simulink model "large_model"  (Simulink 8.0)
settings: Solver=ode45  StartTime=0.0  StopTime=10.0  RelTol=1e-3
(part 2 of 21)

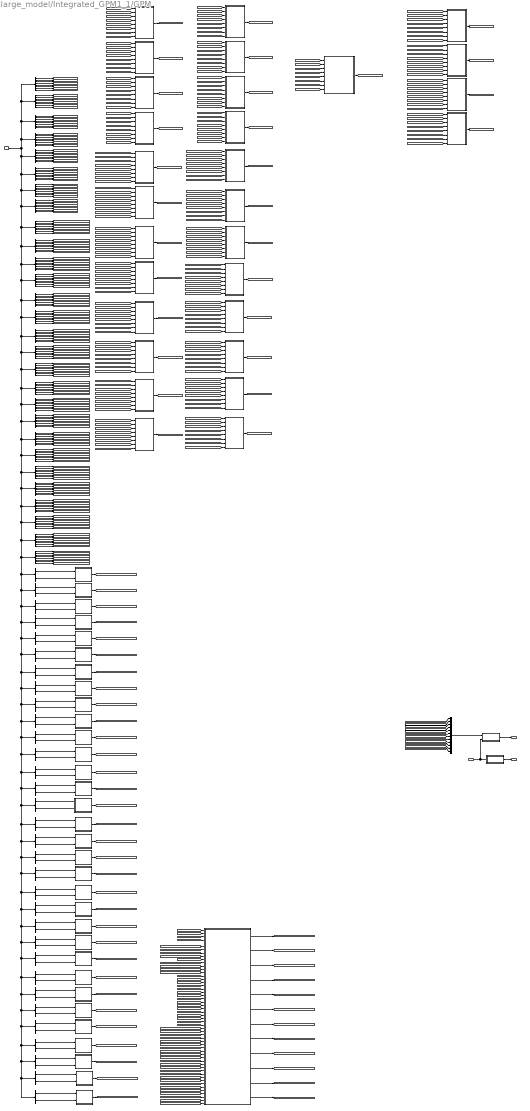
== system large_model/Integrated_GPM1_1/GPM ==
  note: Este componente foi colocado devido a uma limitação do EFTG. Na versão 1.2.1,
      | não é permitido fazer uma conexão direta entre a entrada e a saída.
  From_ES : Inport
  PWR_In : Inport  Port=2
  CAB1_FCC1 : Subsystem
  CAB1_GPM2_fcc_ram_mon_AIL_LIB_dat : BusSelector  OutputSignals=switch1A.cab1_gpm2_chB.CAB1_GPM2_fcc_ram_mon_AIL_LIB_dat,switch1B.cab1_gpm2_chB.CAB1_GPM2_fcc_ram_mon_AIL_LIB_dat
  CAB1_GPM2_fcc_ram_mon_AIL_LOB_dat : BusSelector  OutputSignals=switch1A.cab1_gpm2_chB.CAB1_GPM2_fcc_ram_mon_AIL_LOB_dat,switch1B.cab1_gpm2_chB.CAB1_GPM2_fcc_ram_mon_AIL_LOB_dat
  CAB1_GPM2_fcc_ram_mon_AIL_RIB_dat : BusSelector  OutputSignals=switch1A.cab1_gpm2_chB.CAB1_GPM2_fcc_ram_mon_AIL_RIB_dat,switch1B.cab1_gpm2_chB.CAB1_GPM2_fcc_ram_mon_AIL_RIB_dat
  CAB1_GPM2_fcc_ram_mon_AIL_ROB_dat : BusSelector  OutputSignals=switch1A.cab1_gpm2_chB.CAB1_GPM2_fcc_ram_mon_AIL_ROB_dat,switch1B.cab1_gpm2_chB.CAB1_GPM2_fcc_ram_mon_AIL_ROB_dat
  CAB1_GPM2_fcc_ram_mon_ELEV_LIB_dat : BusSelector  OutputSignals=switch1A.cab1_gpm2_chB.CAB1_GPM2_fcc_ram_mon_ELEV_LIB_dat,switch1B.cab1_gpm2_chB.CAB1_GPM2_fcc_ram_mon_ELEV_LIB_dat
  CAB1_GPM2_fcc_ram_mon_ELEV_LOB_dat : BusSelector  OutputSignals=switch1A.cab1_gpm2_chB.CAB1_GPM2_fcc_ram_mon_ELEV_LOB_dat,switch1B.cab1_gpm2_chB.CAB1_GPM2_fcc_ram_mon_ELEV_LOB_dat
  CAB1_GPM2_fcc_ram_mon_ELEV_RIB_dat : BusSelector  OutputSignals=switch1A.cab1_gpm2_chB.CAB1_GPM2_fcc_ram_mon_ELEV_RIB_dat,switch1B.cab1_gpm2_chB.CAB1_GPM2_fcc_ram_mon_ELEV_RIB_dat
  CAB1_GPM2_fcc_ram_mon_ELEV_ROB_dat : BusSelector  OutputSignals=switch1A.cab1_gpm2_chB.CAB1_GPM2_fcc_ram_mon_ELEV_ROB_dat,switch1B.cab1_gpm2_chB.CAB1_GPM2_fcc_ram_mon_ELEV_ROB_dat
  CAB1_GPM2_fcc_ram_mon_rudder_LWR_dat : BusSelector  OutputSignals=switch1A.cab1_gpm2_chB.CAB1_GPM2_fcc_ram_mon_rudder_LWR_dat,switch1B.cab1_gpm2_chB.CAB1_GPM2_fcc_ram_mon_rudder_LWR_dat
  CAB1_GPM2_fcc_ram_mon_rudder_UPR_dat : BusSelector  OutputSignals=switch1A.cab1_gpm2_chB.CAB1_GPM2_fcc_ram_mon_rudder_UPR_dat,switch1B.cab1_gpm2_chB.CAB1_GPM2_fcc_ram_mon_rudder_UPR_dat
  CAB1_GPM2_pitch_roll_cmd : BusSelector  OutputSignals=switch1A.cab1_gpm2_chB.CAB1_GPM2_pitch_roll_cmd,switch1B.cab1_gpm2_chB.CAB1_GPM2_pitch_roll_cmd
  CAB1_GPM2_yaw_cmd : BusSelector  OutputSignals=switch1A.cab1_gpm2_chB.CAB1_GPM2_yaw_cmd,switch1B.cab1_gpm2_chB.CAB1_GPM2_yaw_cmd
  GPM Hardware : Subsystem
  Outputs : BusCreator  Inputs=12
  PWR : Subsystem
  SCP_CAB1_GPM2_fcc_ram_mon_AIL_LIB : Subsystem
  SCP_CAB1_GPM2_fcc_ram_mon_AIL_LOB : Subsystem
  SCP_CAB1_GPM2_fcc_ram_mon_AIL_RIB : Subsystem
  SCP_CAB1_GPM2_fcc_ram_mon_AIL_ROB : Subsystem
  SCP_CAB1_GPM2_fcc_ram_mon_ELEV_LIB : Subsystem
  SCP_CAB1_GPM2_fcc_ram_mon_ELEV_LOB : Subsystem
  SCP_CAB1_GPM2_fcc_ram_mon_ELEV_RIB : Subsystem
  SCP_CAB1_GPM2_fcc_ram_mon_ELEV_ROB : Subsystem
  SCP_CAB1_GPM2_fcc_ram_mon_rudder_LWR : Subsystem
  SCP_CAB1_GPM2_fcc_ram_mon_rudder_UPR : Subsystem
  SCP_CAB1_GPM2_pitch_roll : Subsystem
  SCP_CAB1_GPM2_yaw : Subsystem
  SCP_ail_REU_LIB_LVDT : Subsystem
  SCP_ail_REU_LIB_mon : Subsystem
  SCP_ail_REU_LOB_LVDT : Subsystem
  SCP_ail_REU_LOB_mon : Subsystem
  SCP_ail_REU_RIB_LVDT : Subsystem
  SCP_ail_REU_RIB_mon : Subsystem
  SCP_ail_REU_ROB_LVDT : Subsystem
  SCP_ail_REU_ROB_mon : Subsystem
  SCP_elev_REU_LIB_LVDT : Subsystem
  SCP_elev_REU_LIB_mon_dat : Subsystem
  SCP_elev_REU_LOB_LVDT : Subsystem
  SCP_elev_REU_LOB_mon : Subsystem
  SCP_elev_REU_RIB_LVDT : Subsystem
  SCP_elev_REU_RIB_mon : Subsystem
  SCP_elev_REU_ROB_LVDT : Subsystem
  SCP_elev_REU_ROB_mon : Subsystem
  SCP_rud_REU1_LVDT : Subsystem
  SCP_rud_REU1_MON : Subsystem
  SCP_rud_REU2_LVDT : Subsystem
  SCP_rud_REU2_MON : Subsystem
  Self_checking_pair_ADS1A : Subsystem
  Self_checking_pair_ADS1B : Subsystem
  Self_checking_pair_ADS2A : Subsystem
  Self_checking_pair_ADS2B : Subsystem
  Self_checking_pair_ADS3A : Subsystem
  Self_checking_pair_ADS3B : Subsystem
  Self_checking_pair_ADS4A : Subsystem
  Self_checking_pair_ADS4B : Subsystem
  Self_checking_pair_PedalYaw1 : Subsystem
  Self_checking_pair_PedalYaw2 : Subsystem
  Self_checking_pair_PedalYaw3 : Subsystem
  Self_checking_pair_PedalYaw4 : Subsystem
  Self_checking_pair_SSC1_pitch1 : Subsystem
  Self_checking_pair_SSC1_pitch2 : Subsystem
  Self_checking_pair_SSC1_pitch3 : Subsystem
  Self_checking_pair_SSC1_pitch4 : Subsystem
  Self_checking_pair_SSC1_roll1 : Subsystem
  Self_checking_pair_SSC1_roll2 : Subsystem
  Self_checking_pair_SSC1_roll3 : Subsystem
  Self_checking_pair_SSC1_roll4 : Subsystem
  Self_checking_pair_SSC2_pitch1 : Subsystem
  Self_checking_pair_SSC2_pitch2 : Subsystem
  Self_checking_pair_SSC2_pitch3 : Subsystem
  Self_checking_pair_SSC2_pitch4 : Subsystem
  Self_checking_pair_SSC2_roll1 : Subsystem
  Self_checking_pair_SSC2_roll2 : Subsystem
  Self_checking_pair_SSC2_roll3 : Subsystem
  Self_checking_pair_SSC2_roll4 : Subsystem
  Voter_ADS : Subsystem
  ads_1A : BusSelector  Description=|  OutputSignals=switch1A.cab2_gpm_IO_chB.ads_1A,switch1A.cab1_gpm_IO_chB.ads_1A,switch1A.switch2A_to_switch.cab3_gpm_IO_chA.ads_1A,switch1A.switch2A_to_switch.cab4_gpm_IO_chA.ads_1A,switch1B.cab2_gpm_IO_chB.ads_1A,switch1B.cab1_gpm_IO_chB.ads_1A,switch1B.switch2B_to_switch.cab4_gpm_IO_chB.ads_1A,switch1B.switch2B_to_switch.cab3_gpm_IO_chB.ads_1A
      | When connected to a line which contains bus signals, this bus selector will select as its outputs all the signals which contain this bus selector name.
      | For example, if you name this bus selector as "sidestick_1_pitch_1", all signals containing this string will be selected as its outputs.
  ads_1B : BusSelector  Description=|  OutputSignals=switch1A.cab2_gpm_IO_chB.ads_1B,switch1A.cab1_gpm_IO_chB.ads_1B,switch1A.switch2A_to_switch.cab3_gpm_IO_chA.ads_1B,switch1A.switch2A_to_switch.cab4_gpm_IO_chA.ads_1B,switch1B.cab2_gpm_IO_chB.ads_1B,switch1B.cab1_gpm_IO_chB.ads_1B,switch1B.switch2B_to_switch.cab4_gpm_IO_chB.ads_1B,switch1B.switch2B_to_switch.cab3_gpm_IO_chB.ads_1B
      | When connected to a line which contains bus signals, this bus selector will select as its outputs all the signals which contain this bus selector name.
      | For example, if you name this bus selector as "sidestick_1_pitch_1", all signals containing this string will be selected as its outputs.
  ads_2A : BusSelector  Description=|  OutputSignals=switch1A.cab2_gpm_IO_chB.ads_2A,switch1A.cab1_gpm_IO_chB.ads_2A,switch1A.switch2A_to_switch.cab3_gpm_IO_chA.ads_2A,switch1A.switch2A_to_switch.cab4_gpm_IO_chA.ads_2A,switch1B.cab2_gpm_IO_chB.ads_2A,switch1B.cab1_gpm_IO_chB.ads_2A,switch1B.switch2B_to_switch.cab4_gpm_IO_chB.ads_2A,switch1B.switch2B_to_switch.cab3_gpm_IO_chB.ads_2A
      | When connected to a line which contains bus signals, this bus selector will select as its outputs all the signals which contain this bus selector name.
      | For example, if you name this bus selector as "sidestick_1_pitch_1", all signals containing this string will be selected as its outputs.
  ads_2B : BusSelector  Description=|  OutputSignals=switch1A.cab2_gpm_IO_chB.ads_2B,switch1A.cab1_gpm_IO_chB.ads_2B,switch1A.switch2A_to_switch.cab3_gpm_IO_chA.ads_2B,switch1A.switch2A_to_switch.cab4_gpm_IO_chA.ads_2B,switch1B.cab2_gpm_IO_chB.ads_2B,switch1B.cab1_gpm_IO_chB.ads_2B,switch1B.switch2B_to_switch.cab4_gpm_IO_chB.ads_2B,switch1B.switch2B_to_switch.cab3_gpm_IO_chB.ads_2B
      | When connected to a line which contains bus signals, this bus selector will select as its outputs all the signals which contain this bus selector name.
      | For example, if you name this bus selector as "sidestick_1_pitch_1", all signals containing this string will be selected as its outputs.
  ads_3A : BusSelector  Description=|  OutputSignals=switch1A.cab2_gpm_IO_chB.ads_3A,switch1A.cab1_gpm_IO_chB.ads_3A,switch1A.switch2A_to_switch.cab3_gpm_IO_chA.ads_3A,switch1A.switch2A_to_switch.cab4_gpm_IO_chA.ads_3A,switch1B.cab2_gpm_IO_chB.ads_3A,switch1B.cab1_gpm_IO_chB.ads_3A,switch1B.switch2B_to_switch.cab4_gpm_IO_chB.ads_3A,switch1B.switch2B_to_switch.cab3_gpm_IO_chB.ads_3A
      | When connected to a line which contains bus signals, this bus selector will select as its outputs all the signals which contain this bus selector name.
      | For example, if you name this bus selector as "sidestick_1_pitch_1", all signals containing this string will be selected as its outputs.
  ads_3B : BusSelector  Description=|  OutputSignals=switch1A.cab2_gpm_IO_chB.ads_3B,switch1A.cab1_gpm_IO_chB.ads_3B,switch1A.switch2A_to_switch.cab3_gpm_IO_chA.ads_3B,switch1A.switch2A_to_switch.cab4_gpm_IO_chA.ads_3B,switch1B.cab2_gpm_IO_chB.ads_3B,switch1B.cab1_gpm_IO_chB.ads_3B,switch1B.switch2B_to_switch.cab4_gpm_IO_chB.ads_3B,switch1B.switch2B_to_switch.cab3_gpm_IO_chB.ads_3B
      | When connected to a line which contains bus signals, this bus selector will select as its outputs all the signals which contain this bus selector name.
      | For example, if you name this bus selector as "sidestick_1_pitch_1", all signals containing this string will be selected as its outputs.
  ads_4A : BusSelector  Description=|  OutputSignals=switch1A.cab2_gpm_IO_chB.ads_4A,switch1A.cab1_gpm_IO_chB.ads_4A,switch1A.switch2A_to_switch.cab3_gpm_IO_chA.ads_4A,switch1A.switch2A_to_switch.cab4_gpm_IO_chA.ads_4A,switch1B.cab2_gpm_IO_chB.ads_4A,switch1B.cab1_gpm_IO_chB.ads_4A,switch1B.switch2B_to_switch.cab4_gpm_IO_chB.ads_4A,switch1B.switch2B_to_switch.cab3_gpm_IO_chB.ads_4A
      | When connected to a line which contains bus signals, this bus selector will select as its outputs all the signals which contain this bus selector name.
      | For example, if you name this bus selector as "sidestick_1_pitch_1", all signals containing this string will be selected as its outputs.
  ads_4B : BusSelector  Description=|  OutputSignals=switch1A.cab2_gpm_IO_chB.ads_4B,switch1A.cab1_gpm_IO_chB.ads_4B,switch1A.switch2A_to_switch.cab3_gpm_IO_chA.ads_4B,switch1A.switch2A_to_switch.cab4_gpm_IO_chA.ads_4B,switch1B.cab2_gpm_IO_chB.ads_4B,switch1B.cab1_gpm_IO_chB.ads_4B,switch1B.switch2B_to_switch.cab4_gpm_IO_chB.ads_4B,switch1B.switch2B_to_switch.cab3_gpm_IO_chB.ads_4B
      | When connected to a line which contains bus signals, this bus selector will select as its outputs all the signals which contain this bus selector name.
      | For example, if you name this bus selector as "sidestick_1_pitch_1", all signals containing this string will be selected as its outputs.
  ail_REU_LIB_LVDT_dat : BusSelector  OutputSignals=switch1A.switch2A_to_switch.REU_to_Switch2A.ail_REU_LIB_LVDT_dat,switch1B.switch2B_to_switch.REU_to_Switch2B.ail_REU_LIB_LVDT_dat
  ail_REU_LIB_mon_dat : BusSelector  OutputSignals=switch1A.switch2A_to_switch.REU_to_Switch2A.ail_REU_LIB_mon_dat,switch1B.switch2B_to_switch.REU_to_Switch2B.ail_REU_LIB_mon_dat
  ail_REU_LOB_LVDT_dat : BusSelector  OutputSignals=switch1A.REU_to_Switch1A.ail_REU_LOB_LVDT_dat,switch1B.REU_to_Switch1B.ail_REU_LOB_LVDT_dat
  ail_REU_LOB_mon_dat : BusSelector  OutputSignals=switch1A.REU_to_Switch1A.ail_REU_LOB_mon_dat,switch1B.REU_to_Switch1B.ail_REU_LOB_mon_dat
  ail_REU_RIB_LVDT_dat : BusSelector  OutputSignals=switch1A.switch2A_to_switch.REU_to_Switch2A.ail_REU_RIB_LVDT_dat,switch1B.switch2B_to_switch.REU_to_Switch2B.ail_REU_RIB_LVDT_dat
  ail_REU_RIB_mon_dat : BusSelector  OutputSignals=switch1A.switch2A_to_switch.REU_to_Switch2A.ail_REU_RIB_mon_dat,switch1B.switch2B_to_switch.REU_to_Switch2B.ail_REU_RIB_mon_dat
  ail_REU_ROB_LVDT_dat : BusSelector  OutputSignals=switch1A.REU_to_Switch1A.ail_REU_ROB_LVDT_dat,switch1B.REU_to_Switch1B.ail_REU_ROB_LVDT_dat
  ail_REU_ROB_mon_dat : BusSelector  OutputSignals=switch1A.REU_to_Switch1A.ail_REU_ROB_mon_dat,switch1B.REU_to_Switch1B.ail_REU_ROB_mon_dat
  elev_REU_LIB_LVDT_dat : BusSelector  OutputSignals=switch1A.switch2A_to_switch.REU_to_Switch2A.elev_REU_LIB_LVDT_dat,switch1B.switch2B_to_switch.REU_to_Switch2B.elev_REU_LIB_LVDT_dat
  elev_REU_LIB_mon_dat : BusSelector  OutputSignals=switch1A.switch2A_to_switch.REU_to_Switch2A.elev_REU_LIB_mon_dat,switch1B.switch2B_to_switch.REU_to_Switch2B.elev_REU_LIB_mon_dat
  elev_REU_LOB_LVDT_dat : BusSelector  OutputSignals=switch1A.REU_to_Switch1A.elev_REU_LOB_LVDT_dat,switch1B.REU_to_Switch1B.elev_REU_LOB_LVDT_dat
  elev_REU_LOB_mon_dat : BusSelector  OutputSignals=switch1A.REU_to_Switch1A.elev_REU_LOB_mon_dat,switch1B.REU_to_Switch1B.elev_REU_LOB_mon_dat
  elev_REU_RIB_LVDT_dat : BusSelector  OutputSignals=switch1A.switch2A_to_switch.REU_to_Switch2A.elev_REU_RIB_LVDT_dat,switch1B.switch2B_to_switch.REU_to_Switch2B.elev_REU_RIB_LVDT_dat
  elev_REU_RIB_mon_dat : BusSelector  OutputSignals=switch1A.switch2A_to_switch.REU_to_Switch2A.elev_REU_RIB_mon_dat,switch1B.switch2B_to_switch.REU_to_Switch2B.elev_REU_RIB_mon_dat
  elev_REU_ROB_LVDT_dat : BusSelector  OutputSignals=switch1A.REU_to_Switch1A.elev_REU_ROB_LVDT_dat,switch1B.REU_to_Switch1B.elev_REU_ROB_LVDT_dat
  elev_REU_ROB_mon_dat : BusSelector  OutputSignals=switch1A.REU_to_Switch1A.elev_REU_ROB_mon_dat,switch1B.REU_to_Switch1B.elev_REU_ROB_mon_dat
  pedal_yaw_1 : BusSelector  Description=|  OutputSignals=switch1A.cab2_gpm_IO_chB.rdc_4002_1.pedal_yaw_1,switch1A.cab1_gpm_IO_chB.rdc_4002_1.pedal_yaw_1,switch1A.switch2A_to_switch.cab3_gpm_IO_chA.rdc_4002_1.pedal_yaw_1,switch1A.switch2A_to_switch.cab4_gpm_IO_chA.rdc_4002_1.pedal_yaw_1,switch1B.cab2_gpm_IO_chB.rdc_4002_1.pedal_yaw_1,switch1B.cab1_gpm_IO_chB.rdc_4002_1.pedal_yaw_1,switch1B.switch2B_to_switch.cab4_gpm_IO_chB.rdc_4002_1.pedal_yaw_1,switch1B.switch2B_to_switch.cab3_gpm_IO_chB.rdc_4002_1.pedal_yaw_1
      | When connected to a line which contains bus signals, this bus selector will select as its outputs all the signals which contain this bus selector name.
      | For example, if you name this bus selector as "sidestick_1_pitch_1", all signals containing this string will be selected as its outputs.
  pedal_yaw_2 : BusSelector  Description=|  OutputSignals=switch1A.cab2_gpm_IO_chB.rdc_4002_2.pedal_yaw_2,switch1A.cab1_gpm_IO_chB.rdc_4002_2.pedal_yaw_2,switch1A.switch2A_to_switch.cab3_gpm_IO_chA.rdc_4002_2.pedal_yaw_2,switch1A.switch2A_to_switch.cab4_gpm_IO_chA.rdc_4002_2.pedal_yaw_2,switch1B.cab2_gpm_IO_chB.rdc_4002_2.pedal_yaw_2,switch1B.cab1_gpm_IO_chB.rdc_4002_2.pedal_yaw_2,switch1B.switch2B_to_switch.cab4_gpm_IO_chB.rdc_4002_2.pedal_yaw_2,switch1B.switch2B_to_switch.cab3_gpm_IO_chB.rdc_4002_2.pedal_yaw_2
      | When connected to a line which contains bus signals, this bus selector will select as its outputs all the signals which contain this bus selector name.
      | For example, if you name this bus selector as "sidestick_1_pitch_1", all signals containing this string will be selected as its outputs.
  pedal_yaw_3 : BusSelector  Description=|  OutputSignals=switch1A.cab2_gpm_IO_chB.rdc_4002_3.pedal_yaw_3,switch1A.cab1_gpm_IO_chB.rdc_4002_3.pedal_yaw_3,switch1A.switch2A_to_switch.cab3_gpm_IO_chA.rdc_4002_3.pedal_yaw_3,switch1A.switch2A_to_switch.cab4_gpm_IO_chA.rdc_4002_3.pedal_yaw_3,switch1B.cab2_gpm_IO_chB.rdc_4002_3.pedal_yaw_3,switch1B.cab1_gpm_IO_chB.rdc_4002_3.pedal_yaw_3,switch1B.switch2B_to_switch.cab4_gpm_IO_chB.rdc_4002_3.pedal_yaw_3,switch1B.switch2B_to_switch.cab3_gpm_IO_chB.rdc_4002_3.pedal_yaw_3
      | When connected to a line which contains bus signals, this bus selector will select as its outputs all the signals which contain this bus selector name.
      | For example, if you name this bus selector as "sidestick_1_pitch_1", all signals containing this string will be selected as its outputs.
  pedal_yaw_4 : BusSelector  Description=|  OutputSignals=switch1A.cab2_gpm_IO_chB.rdc_4002_4.pedal_yaw_4,switch1A.cab1_gpm_IO_chB.rdc_4002_4.pedal_yaw_4,switch1A.switch2A_to_switch.cab3_gpm_IO_chA.rdc_4002_4.pedal_yaw_4,switch1A.switch2A_to_switch.cab4_gpm_IO_chA.rdc_4002_4.pedal_yaw_4,switch1B.cab2_gpm_IO_chB.rdc_4002_4.pedal_yaw_4,switch1B.cab1_gpm_IO_chB.rdc_4002_4.pedal_yaw_4,switch1B.switch2B_to_switch.cab4_gpm_IO_chB.rdc_4002_4.pedal_yaw_4,switch1B.switch2B_to_switch.cab3_gpm_IO_chB.rdc_4002_4.pedal_yaw_4
      | When connected to a line which contains bus signals, this bus selector will select as its outputs all the signals which contain this bus selector name.
      | For example, if you name this bus selector as "sidestick_1_pitch_1", all signals containing this string will be selected as its outputs.
  rud_REU1_LVDT_dat : BusSelector  OutputSignals=switch1A.switch2A_to_switch.REU_to_Switch2A.rud_REU1_LVDT_dat,switch1B.switch2B_to_switch.REU_to_Switch2B.rud_REU1_LVDT_dat
  rud_REU1_MON_dat : BusSelector  OutputSignals=switch1A.switch2A_to_switch.REU_to_Switch2A.rud_REU1_MON_dat,switch1B.switch2B_to_switch.REU_to_Switch2B.rud_REU1_MON_dat
  rud_REU2_LVDT_dat : BusSelector  OutputSignals=switch1A.REU_to_Switch1A.rud_REU2_LVDT_dat,switch1B.REU_to_Switch1B.rud_REU2_LVDT_dat
  rud_REU2_MON_dat : BusSelector  OutputSignals=switch1A.REU_to_Switch1A.rud_REU2_MON_dat,switch1B.REU_to_Switch1B.rud_REU2_MON_dat
  sidestick1_pitch_1 : BusSelector  OutputSignals=switch1A.cab2_gpm_IO_chB.rdc_4002_1.sidesticks.sidestick1_pitch_1,switch1A.cab1_gpm_IO_chB.rdc_4002_1.sidesticks.sidestick1_pitch_1,switch1A.switch2A_to_switch.cab3_gpm_IO_chA.rdc_4002_1.sidesticks.sidestick1_pitch_1,switch1A.switch2A_to_switch.cab4_gpm_IO_chA.rdc_4002_1.sidesticks.sidestick1_pitch_1,switch1B.cab2_gpm_IO_chB.rdc_4002_1.sidesticks.sidestick1_pitch_1,switch1B.cab1_gpm_IO_chB.rdc_4002_1.sidesticks.sidestick1_pitch_1,switch1B.switch2B_to_switch.cab4_gpm_IO_chB.rdc_4002_1.sidesticks.sidestick1_pitch_1,switch1B.switch2B_to_switch.cab3_gpm_IO_chB.rdc_4002_1.sidesticks.sidestick1_pitch_1
  sidestick1_pitch_2 : BusSelector  OutputSignals=switch1A.cab2_gpm_IO_chB.rdc_4002_2.sidesticks.sidestick1_pitch_2,switch1A.cab1_gpm_IO_chB.rdc_4002_2.sidesticks.sidestick1_pitch_2,switch1A.switch2A_to_switch.cab3_gpm_IO_chA.rdc_4002_2.sidesticks.sidestick1_pitch_2,switch1A.switch2A_to_switch.cab4_gpm_IO_chA.rdc_4002_2.sidesticks.sidestick1_pitch_2,switch1B.cab2_gpm_IO_chB.rdc_4002_2.sidesticks.sidestick1_pitch_2,switch1B.cab1_gpm_IO_chB.rdc_4002_2.sidesticks.sidestick1_pitch_2,switch1B.switch2B_to_switch.cab4_gpm_IO_chB.rdc_4002_2.sidesticks.sidestick1_pitch_2,switch1B.switch2B_to_switch.cab3_gpm_IO_chB.rdc_4002_2.sidesticks.sidestick1_pitch_2
  sidestick1_pitch_3 : BusSelector  OutputSignals=switch1A.cab2_gpm_IO_chB.rdc_4002_3.sidesticks.sidestick1_pitch_3,switch1A.cab1_gpm_IO_chB.rdc_4002_3.sidesticks.sidestick1_pitch_3,switch1A.switch2A_to_switch.cab3_gpm_IO_chA.rdc_4002_3.sidesticks.sidestick1_pitch_3,switch1A.switch2A_to_switch.cab4_gpm_IO_chA.rdc_4002_3.sidesticks.sidestick1_pitch_3,switch1B.cab2_gpm_IO_chB.rdc_4002_3.sidesticks.sidestick1_pitch_3,switch1B.cab1_gpm_IO_chB.rdc_4002_3.sidesticks.sidestick1_pitch_3,switch1B.switch2B_to_switch.cab4_gpm_IO_chB.rdc_4002_3.sidesticks.sidestick1_pitch_3,switch1B.switch2B_to_switch.cab3_gpm_IO_chB.rdc_4002_3.sidesticks.sidestick1_pitch_3
  sidestick1_pitch_4 : BusSelector  OutputSignals=switch1A.cab2_gpm_IO_chB.rdc_4002_4.sidesticks.sidestick1_pitch_4,switch1A.cab1_gpm_IO_chB.rdc_4002_4.sidesticks.sidestick1_pitch_4,switch1A.switch2A_to_switch.cab3_gpm_IO_chA.rdc_4002_4.sidesticks.sidestick1_pitch_4,switch1A.switch2A_to_switch.cab4_gpm_IO_chA.rdc_4002_4.sidesticks.sidestick1_pitch_4,switch1B.cab2_gpm_IO_chB.rdc_4002_4.sidesticks.sidestick1_pitch_4,switch1B.cab1_gpm_IO_chB.rdc_4002_4.sidesticks.sidestick1_pitch_4,switch1B.switch2B_to_switch.cab4_gpm_IO_chB.rdc_4002_4.sidesticks.sidestick1_pitch_4,switch1B.switch2B_to_switch.cab3_gpm_IO_chB.rdc_4002_4.sidesticks.sidestick1_pitch_4
  sidestick1_roll_1 : BusSelector  OutputSignals=switch1A.cab2_gpm_IO_chB.rdc_4002_1.sidesticks.sidestick1_roll_1,switch1A.cab1_gpm_IO_chB.rdc_4002_1.sidesticks.sidestick1_roll_1,switch1A.switch2A_to_switch.cab3_gpm_IO_chA.rdc_4002_1.sidesticks.sidestick1_roll_1,switch1A.switch2A_to_switch.cab4_gpm_IO_chA.rdc_4002_1.sidesticks.sidestick1_roll_1,switch1B.cab2_gpm_IO_chB.rdc_4002_1.sidesticks.sidestick1_roll_1,switch1B.cab1_gpm_IO_chB.rdc_4002_1.sidesticks.sidestick1_roll_1,switch1B.switch2B_to_switch.cab4_gpm_IO_chB.rdc_4002_1.sidesticks.sidestick1_roll_1,switch1B.switch2B_to_switch.cab3_gpm_IO_chB.rdc_4002_1.sidesticks.sidestick1_roll_1
  sidestick1_roll_2 : BusSelector  OutputSignals=switch1A.cab2_gpm_IO_chB.rdc_4002_2.sidesticks.sidestick1_roll_2,switch1A.cab1_gpm_IO_chB.rdc_4002_2.sidesticks.sidestick1_roll_2,switch1A.switch2A_to_switch.cab3_gpm_IO_chA.rdc_4002_2.sidesticks.sidestick1_roll_2,switch1A.switch2A_to_switch.cab4_gpm_IO_chA.rdc_4002_2.sidesticks.sidestick1_roll_2,switch1B.cab2_gpm_IO_chB.rdc_4002_2.sidesticks.sidestick1_roll_2,switch1B.cab1_gpm_IO_chB.rdc_4002_2.sidesticks.sidestick1_roll_2,switch1B.switch2B_to_switch.cab4_gpm_IO_chB.rdc_4002_2.sidesticks.sidestick1_roll_2,switch1B.switch2B_to_switch.cab3_gpm_IO_chB.rdc_4002_2.sidesticks.sidestick1_roll_2
  sidestick1_roll_3 : BusSelector  OutputSignals=switch1A.cab2_gpm_IO_chB.rdc_4002_3.sidesticks.sidestick1_roll_3,switch1A.cab1_gpm_IO_chB.rdc_4002_3.sidesticks.sidestick1_roll_3,switch1A.switch2A_to_switch.cab3_gpm_IO_chA.rdc_4002_3.sidesticks.sidestick1_roll_3,switch1A.switch2A_to_switch.cab4_gpm_IO_chA.rdc_4002_3.sidesticks.sidestick1_roll_3,switch1B.cab2_gpm_IO_chB.rdc_4002_3.sidesticks.sidestick1_roll_3,switch1B.cab1_gpm_IO_chB.rdc_4002_3.sidesticks.sidestick1_roll_3,switch1B.switch2B_to_switch.cab4_gpm_IO_chB.rdc_4002_3.sidesticks.sidestick1_roll_3,switch1B.switch2B_to_switch.cab3_gpm_IO_chB.rdc_4002_3.sidesticks.sidestick1_roll_3
  sidestick1_roll_4 : BusSelector  OutputSignals=switch1A.cab2_gpm_IO_chB.rdc_4002_4.sidesticks.sidestick1_roll_4,switch1A.cab1_gpm_IO_chB.rdc_4002_4.sidesticks.sidestick1_roll_4,switch1A.switch2A_to_switch.cab3_gpm_IO_chA.rdc_4002_4.sidesticks.sidestick1_roll_4,switch1A.switch2A_to_switch.cab4_gpm_IO_chA.rdc_4002_4.sidesticks.sidestick1_roll_4,switch1B.cab2_gpm_IO_chB.rdc_4002_4.sidesticks.sidestick1_roll_4,switch1B.cab1_gpm_IO_chB.rdc_4002_4.sidesticks.sidestick1_roll_4,switch1B.switch2B_to_switch.cab4_gpm_IO_chB.rdc_4002_4.sidesticks.sidestick1_roll_4,switch1B.switch2B_to_switch.cab3_gpm_IO_chB.rdc_4002_4.sidesticks.sidestick1_roll_4
  sidestick2_pitch_1 : BusSelector  OutputSignals=switch1A.cab2_gpm_IO_chB.rdc_4002_1.sidesticks.sidestick2_pitch_1,switch1A.cab1_gpm_IO_chB.rdc_4002_1.sidesticks.sidestick2_pitch_1,switch1A.switch2A_to_switch.cab3_gpm_IO_chA.rdc_4002_1.sidesticks.sidestick2_pitch_1,switch1A.switch2A_to_switch.cab4_gpm_IO_chA.rdc_4002_1.sidesticks.sidestick2_pitch_1,switch1B.cab2_gpm_IO_chB.rdc_4002_1.sidesticks.sidestick2_pitch_1,switch1B.cab1_gpm_IO_chB.rdc_4002_1.sidesticks.sidestick2_pitch_1,switch1B.switch2B_to_switch.cab4_gpm_IO_chB.rdc_4002_1.sidesticks.sidestick2_pitch_1,switch1B.switch2B_to_switch.cab3_gpm_IO_chB.rdc_4002_1.sidesticks.sidestick2_pitch_1
  sidestick2_pitch_2 : BusSelector  OutputSignals=switch1A.cab2_gpm_IO_chB.rdc_4002_2.sidesticks.sidestick2_pitch_2,switch1A.cab1_gpm_IO_chB.rdc_4002_2.sidesticks.sidestick2_pitch_2,switch1A.switch2A_to_switch.cab3_gpm_IO_chA.rdc_4002_2.sidesticks.sidestick2_pitch_2,switch1A.switch2A_to_switch.cab4_gpm_IO_chA.rdc_4002_2.sidesticks.sidestick2_pitch_2,switch1B.cab2_gpm_IO_chB.rdc_4002_2.sidesticks.sidestick2_pitch_2,switch1B.cab1_gpm_IO_chB.rdc_4002_2.sidesticks.sidestick2_pitch_2,switch1B.switch2B_to_switch.cab4_gpm_IO_chB.rdc_4002_2.sidesticks.sidestick2_pitch_2,switch1B.switch2B_to_switch.cab3_gpm_IO_chB.rdc_4002_2.sidesticks.sidestick2_pitch_2
  sidestick2_pitch_3 : BusSelector  OutputSignals=switch1A.cab2_gpm_IO_chB.rdc_4002_3.sidesticks.sidestick2_pitch_3,switch1A.cab1_gpm_IO_chB.rdc_4002_3.sidesticks.sidestick2_pitch_3,switch1A.switch2A_to_switch.cab3_gpm_IO_chA.rdc_4002_3.sidesticks.sidestick2_pitch_3,switch1A.switch2A_to_switch.cab4_gpm_IO_chA.rdc_4002_3.sidesticks.sidestick2_pitch_3,switch1B.cab2_gpm_IO_chB.rdc_4002_3.sidesticks.sidestick2_pitch_3,switch1B.cab1_gpm_IO_chB.rdc_4002_3.sidesticks.sidestick2_pitch_3,switch1B.switch2B_to_switch.cab4_gpm_IO_chB.rdc_4002_3.sidesticks.sidestick2_pitch_3,switch1B.switch2B_to_switch.cab3_gpm_IO_chB.rdc_4002_3.sidesticks.sidestick2_pitch_3
  sidestick2_pitch_4 : BusSelector  OutputSignals=switch1A.cab2_gpm_IO_chB.rdc_4002_4.sidesticks.sidestick2_pitch_4,switch1A.cab1_gpm_IO_chB.rdc_4002_4.sidesticks.sidestick2_pitch_4,switch1A.switch2A_to_switch.cab3_gpm_IO_chA.rdc_4002_4.sidesticks.sidestick2_pitch_4,switch1A.switch2A_to_switch.cab4_gpm_IO_chA.rdc_4002_4.sidesticks.sidestick2_pitch_4,switch1B.cab2_gpm_IO_chB.rdc_4002_4.sidesticks.sidestick2_pitch_4,switch1B.cab1_gpm_IO_chB.rdc_4002_4.sidesticks.sidestick2_pitch_4,switch1B.switch2B_to_switch.cab4_gpm_IO_chB.rdc_4002_4.sidesticks.sidestick2_pitch_4,switch1B.switch2B_to_switch.cab3_gpm_IO_chB.rdc_4002_4.sidesticks.sidestick2_pitch_4
  sidestick2_roll_1 : BusSelector  OutputSignals=switch1A.cab2_gpm_IO_chB.rdc_4002_1.sidesticks.sidestick2_roll_1,switch1A.cab1_gpm_IO_chB.rdc_4002_1.sidesticks.sidestick2_roll_1,switch1A.switch2A_to_switch.cab3_gpm_IO_chA.rdc_4002_1.sidesticks.sidestick2_roll_1,switch1A.switch2A_to_switch.cab4_gpm_IO_chA.rdc_4002_1.sidesticks.sidestick2_roll_1,switch1B.cab2_gpm_IO_chB.rdc_4002_1.sidesticks.sidestick2_roll_1,switch1B.cab1_gpm_IO_chB.rdc_4002_1.sidesticks.sidestick2_roll_1,switch1B.switch2B_to_switch.cab4_gpm_IO_chB.rdc_4002_1.sidesticks.sidestick2_roll_1,switch1B.switch2B_to_switch.cab3_gpm_IO_chB.rdc_4002_1.sidesticks.sidestick2_roll_1
  sidestick2_roll_2 : BusSelector  OutputSignals=switch1A.cab2_gpm_IO_chB.rdc_4002_2.sidesticks.sidestick2_roll_2,switch1A.cab1_gpm_IO_chB.rdc_4002_2.sidesticks.sidestick2_roll_2,switch1A.switch2A_to_switch.cab3_gpm_IO_chA.rdc_4002_2.sidesticks.sidestick2_roll_2,switch1A.switch2A_to_switch.cab4_gpm_IO_chA.rdc_4002_2.sidesticks.sidestick2_roll_2,switch1B.cab2_gpm_IO_chB.rdc_4002_2.sidesticks.sidestick2_roll_2,switch1B.cab1_gpm_IO_chB.rdc_4002_2.sidesticks.sidestick2_roll_2,switch1B.switch2B_to_switch.cab4_gpm_IO_chB.rdc_4002_2.sidesticks.sidestick2_roll_2,switch1B.switch2B_to_switch.cab3_gpm_IO_chB.rdc_4002_2.sidesticks.sidestick2_roll_2
  sidestick2_roll_3 : BusSelector  OutputSignals=switch1A.cab2_gpm_IO_chB.rdc_4002_3.sidesticks.sidestick2_roll_3,switch1A.cab1_gpm_IO_chB.rdc_4002_3.sidesticks.sidestick2_roll_3,switch1A.switch2A_to_switch.cab3_gpm_IO_chA.rdc_4002_3.sidesticks.sidestick2_roll_3,switch1A.switch2A_to_switch.cab4_gpm_IO_chA.rdc_4002_3.sidesticks.sidestick2_roll_3,switch1B.cab2_gpm_IO_chB.rdc_4002_3.sidesticks.sidestick2_roll_3,switch1B.cab1_gpm_IO_chB.rdc_4002_3.sidesticks.sidestick2_roll_3,switch1B.switch2B_to_switch.cab4_gpm_IO_chB.rdc_4002_3.sidesticks.sidestick2_roll_3,switch1B.switch2B_to_switch.cab3_gpm_IO_chB.rdc_4002_3.sidesticks.sidestick2_roll_3
  sidestick2_roll_4 : BusSelector  OutputSignals=switch1A.cab2_gpm_IO_chB.rdc_4002_4.sidesticks.sidestick2_roll_4,switch1A.cab1_gpm_IO_chB.rdc_4002_4.sidesticks.sidestick2_roll_4,switch1A.switch2A_to_switch.cab3_gpm_IO_chA.rdc_4002_4.sidesticks.sidestick2_roll_4,switch1A.switch2A_to_switch.cab4_gpm_IO_chA.rdc_4002_4.sidesticks.sidestick2_roll_4,switch1B.cab2_gpm_IO_chB.rdc_4002_4.sidesticks.sidestick2_roll_4,switch1B.cab1_gpm_IO_chB.rdc_4002_4.sidesticks.sidestick2_roll_4,switch1B.switch2B_to_switch.cab4_gpm_IO_chB.rdc_4002_4.sidesticks.sidestick2_roll_4,switch1B.switch2B_to_switch.cab3_gpm_IO_chB.rdc_4002_4.sidesticks.sidestick2_roll_4
  To_ES : Outport
  PWR_Out : Outport  Port=2
  wires:
  PWR/1(Out1) -> PWR_Out/1
  GPM Hardware/1(Out) -> To_ES/1
  PWR_In/1 -> PWR/1(In1), GPM Hardware/2(PWR_In)
  Outputs/1 -> GPM Hardware/1(Data)
  Voter_ADS/1(ADS) -> [ads_dat]
  [ads_dat] -> CAB1_FCC1/10(ads_dat)
  [sidestick1Pitch2_cmd] -> CAB1_FCC1/16(sidestick1Pitch2_cmd)
  [sidestick1Pitch3_cmd] -> CAB1_FCC1/17(sidestick1Pitch3_cmd)
  [sidestick1Pitch4_cmd] -> CAB1_FCC1/18(sidestick1Pitch4_cmd)
  [sidestick1Pitch1_cmd] -> CAB1_FCC1/15(sidestick1Pitch1_cmd)
  [sidestick1Roll1_cmd] -> CAB1_FCC1/19(sidestick1Roll1_cmd)
  [sidestick1Roll2_cmd] -> CAB1_FCC1/20(sidestick1Roll2_cmd)
  [sidestick1Roll3_cmd] -> CAB1_FCC1/21(sidestick1Roll3_cmd)
  [PedalYaw1_cmd] -> CAB1_FCC1/1(PedalYaw1_cmd)
  [sidestick2Roll4_cmd] -> CAB1_FCC1/30(sidestick2Roll4_cmd)
  [sidestick2Roll3_cmd] -> CAB1_FCC1/29(sidestick2Roll3_cmd)
  [sidestick2Pitch3_cmd] -> CAB1_FCC1/25(sidestick2Pitch3_cmd)
  [sidestick2Pitch2_cmd] -> CAB1_FCC1/24(sidestick2Pitch2_cmd)
  [sidestick2Pitch1_cmd] -> CAB1_FCC1/23(sidestick2Pitch1_cmd)
  [PedalYaw4_cmd] -> CAB1_FCC1/4(PedalYaw4_cmd)
  [PedalYaw3_cmd] -> CAB1_FCC1/3(PedalYaw3_cmd)
  [PedalYaw2_cmd] -> CAB1_FCC1/2(PedalYaw2_cmd)
  [sidestick1Roll4_cmd] -> CAB1_FCC1/22(sidestick1Roll4_cmd)
  [sidestick2Roll2_cmd] -> CAB1_FCC1/28(sidestick2Roll2_cmd)
  [sidestick2Roll1_cmd] -> CAB1_FCC1/27(sidestick2Roll1_cmd)
  [sidestick2Pitch4_cmd] -> CAB1_FCC1/26(sidestick2Pitch4_cmd)
  Self_checking_pair_ADS1A/1(Out) -> [Self_checking_pair_ADS1A_1_]
  [Self_checking_pair_ADS1A_1_] -> Voter_ADS/1(ADS1A)
  Self_checking_pair_ADS2B/1(Out) -> [Self_checking_pair_ADS2B_1_]
  [Self_checking_pair_ADS2B_1_] -> Voter_ADS/4(ADS2B)
  Self_checking_pair_ADS2A/1(Out) -> [Self_checking_pair_ADS2A_1_]
  [Self_checking_pair_ADS2A_1_] -> Voter_ADS/3(ADS2A)
  Self_checking_pair_ADS1B/1(Out) -> [Self_checking_pair_ADS1B_1_]
  [Self_checking_pair_ADS1B_1_] -> Voter_ADS/2(ADS1B)
  Self_checking_pair_ADS4B/1(Out) -> [Self_checking_pair_ADS4B_1_]
  [Self_checking_pair_ADS4B_1_] -> Voter_ADS/8(ADS4B)
  [Self_checking_pair_ADS4A_1_] -> Voter_ADS/7(ADS4A)
  Self_checking_pair_ADS3B/1(Out) -> [Self_checking_pair_ADS3B_1_]
  [Self_checking_pair_ADS3B_1_] -> Voter_ADS/6(ADS3B)
  [Self_checking_pair_ADS3A_1_] -> Voter_ADS/5(ADS3A)
  Self_checking_pair_ADS3A/1(Out) -> [Self_checking_pair_ADS3A_1_]
  Self_checking_pair_ADS4A/1(Out) -> [Self_checking_pair_ADS4A_1_]
  Self_checking_pair_PedalYaw1/1(Out) -> [PedalYaw1_cmd]
  Self_checking_pair_PedalYaw2/1(Out) -> [PedalYaw2_cmd]
  Self_checking_pair_PedalYaw3/1(Out) -> [PedalYaw3_cmd]
  Self_checking_pair_PedalYaw4/1(Out) -> [PedalYaw4_cmd]
  From_ES/1 -> ads_1A/1, ads_1B/1, ads_2A/1, ads_2B/1, sidestick1_pitch_2/1, sidestick1_pitch_3/1, sidestick2_pitch_1/1, sidestick2_pitch_2/1, sidestick2_pitch_3/1, sidestick2_pitch_4/1, sidestick1_roll_1/1, sidestick1_roll_2/1, sidestick1_roll_3/1, sidestick1_roll_4/1, sidestick2_roll_1/1, sidestick2_roll_2/1, sidestick2_roll_3/1, sidestick2_roll_4/1, ail_REU_LIB_LVDT_dat/1, ail_REU_LIB_mon_dat/1, ail_REU_LOB_LVDT_dat/1, ail_REU_LOB_mon_dat/1, ail_REU_RIB_LVDT_dat/1, ail_REU_RIB_mon_dat/1, ail_REU_ROB_LVDT_dat/1, ail_REU_ROB_mon_dat/1, elev_REU_LIB_LVDT_dat/1, elev_REU_LIB_mon_dat/1, elev_REU_LOB_LVDT_dat/1, elev_REU_LOB_mon_dat/1, rud_REU1_LVDT_dat/1, rud_REU1_MON_dat/1, rud_REU2_LVDT_dat/1, rud_REU2_MON_dat/1, elev_REU_RIB_mon_dat/1, elev_REU_ROB_LVDT_dat/1, elev_REU_ROB_mon_dat/1, CAB1_GPM2_yaw_cmd/1, CAB1_GPM2_pitch_roll_cmd/1, CAB1_GPM2_fcc_ram_mon_rudder_LWR_dat/1, CAB1_GPM2_fcc_ram_mon_rudder_UPR_dat/1, CAB1_GPM2_fcc_ram_mon_AIL_LIB_dat/1, CAB1_GPM2_fcc_ram_mon_AIL_LOB_dat/1, CAB1_GPM2_fcc_ram_mon_AIL_RIB_dat/1, CAB1_GPM2_fcc_ram_mon_AIL_ROB_dat/1, CAB1_GPM2_fcc_ram_mon_ELEV_LIB_dat/1, CAB1_GPM2_fcc_ram_mon_ELEV_LOB_dat/1, CAB1_GPM2_fcc_ram_mon_ELEV_RIB_dat/1, CAB1_GPM2_fcc_ram_mon_ELEV_ROB_dat/1, elev_REU_RIB_LVDT_dat/1, sidestick1_pitch_4/1, sidestick1_pitch_1/1, pedal_yaw_4/1, pedal_yaw_3/1, pedal_yaw_2/1, pedal_yaw_1/1, ads_4B/1, ads_4A/1, ads_3B/1, ads_3A/1
  ads_4B/8 -> [ADS4B_8_ads_4B]  "<ads_4B>"
  [ADS4B_8_ads_4B] -> Self_checking_pair_ADS4B/8(In8)
  ads_4B/7 -> [ADS4B_7_ads_4B]  "<ads_4B>"
  [ADS4B_7_ads_4B] -> Self_checking_pair_ADS4B/7(In7)
  ads_4B/6 -> [ADS4B_6_ads_4B]  "<ads_4B>"
  [ADS4B_6_ads_4B] -> Self_checking_pair_ADS4B/6(In6)
  ads_4B/5 -> [ADS4B_5_ads_4B]  "<ads_4B>"
  [ADS4B_5_ads_4B] -> Self_checking_pair_ADS4B/5(In5)
  ads_4B/4 -> [ADS4B_4_ads_4B]  "<ads_4B>"
  [ADS4B_4_ads_4B] -> Self_checking_pair_ADS4B/4(In4)
  ads_4B/3 -> [ADS4B_3_ads_4B]  "<ads_4B>"
  [ADS4B_3_ads_4B] -> Self_checking_pair_ADS4B/3(In3)
  ads_4B/2 -> [ADS4B_2_ads_4B]  "<ads_4B>"
  [ADS4B_2_ads_4B] -> Self_checking_pair_ADS4B/2(In2)
  ads_4B/1 -> [ADS4B_1_ads_4B]  "<ads_4B>"
  [ADS4B_1_ads_4B] -> Self_checking_pair_ADS4B/1(In1)
  ads_4A/8 -> [ADS4A_8_ads_4A]  "<ads_4A>"
  [ADS4A_8_ads_4A] -> Self_checking_pair_ADS4A/8(In8)
  ads_4A/7 -> [ADS4A_7_ads_4A]  "<ads_4A>"
  [ADS4A_7_ads_4A] -> Self_checking_pair_ADS4A/7(In7)
  ads_4A/6 -> [ADS4A_6_ads_4A]  "<ads_4A>"
  [ADS4A_6_ads_4A] -> Self_checking_pair_ADS4A/6(In6)
  ads_4A/5 -> [ADS4A_5_ads_4A]  "<ads_4A>"
  [ADS4A_5_ads_4A] -> Self_checking_pair_ADS4A/5(In5)
  ads_4A/4 -> [ADS4A_4_ads_4A]  "<ads_4A>"
  [ADS4A_4_ads_4A] -> Self_checking_pair_ADS4A/4(In4)
  ads_4A/3 -> [ADS4A_3_ads_4A]  "<ads_4A>"
  [ADS4A_3_ads_4A] -> Self_checking_pair_ADS4A/3(In3)
  ads_4A/2 -> [ADS4A_2_ads_4A]  "<ads_4A>"
  [ADS4A_2_ads_4A] -> Self_checking_pair_ADS4A/2(In2)
  ads_4A/1 -> [ADS4A_1_ads_4A]  "<ads_4A>"
  [ADS4A_1_ads_4A] -> Self_checking_pair_ADS4A/1(In1)
  ads_3B/8 -> [ADS3B_8_ads_3B]  "<ads_3B>"
  [ADS3B_8_ads_3B] -> Self_checking_pair_ADS3B/8(In8)
  ads_3B/7 -> [ADS3B_7_ads_3B]  "<ads_3B>"
  [ADS3B_7_ads_3B] -> Self_checking_pair_ADS3B/7(In7)
  ads_3B/6 -> [ADS3B_6_ads_3B]  "<ads_3B>"
  [ADS3B_6_ads_3B] -> Self_checking_pair_ADS3B/6(In6)
  ads_3B/5 -> [ADS3B_5_ads_3B]  "<ads_3B>"
  [ADS3B_5_ads_3B] -> Self_checking_pair_ADS3B/5(In5)
  ads_3B/4 -> [ADS3B_4_ads_3B]  "<ads_3B>"
  [ADS3B_4_ads_3B] -> Self_checking_pair_ADS3B/4(In4)
  ads_3B/3 -> [ADS3B_3_ads_3B]  "<ads_3B>"
  [ADS3B_3_ads_3B] -> Self_checking_pair_ADS3B/3(In3)
  ads_3B/2 -> [ADS3B_2_ads_3B]  "<ads_3B>"
  [ADS3B_2_ads_3B] -> Self_checking_pair_ADS3B/2(In2)
  ads_3B/1 -> [ADS3B_1_ads_3B]  "<ads_3B>"
  [ADS3B_1_ads_3B] -> Self_checking_pair_ADS3B/1(In1)
  ads_3A/8 -> [ADS3A_8_ads_3A]  "<ads_3A>"
  [ADS3A_8_ads_3A] -> Self_checking_pair_ADS3A/8(In8)
  ads_3A/7 -> [ADS3A_7_ads_3A]  "<ads_3A>"
  [ADS3A_7_ads_3A] -> Self_checking_pair_ADS3A/7(In7)
  ads_3A/6 -> [ADS3A_6_ads_3A]  "<ads_3A>"
  [ADS3A_6_ads_3A] -> Self_checking_pair_ADS3A/6(In6)
  ads_3A/5 -> [ADS3A_5_ads_3A]  "<ads_3A>"
  [ADS3A_5_ads_3A] -> Self_checking_pair_ADS3A/5(In5)
  ads_3A/4 -> [ADS3A_4_ads_3A]  "<ads_3A>"
  [ADS3A_4_ads_3A] -> Self_checking_pair_ADS3A/4(In4)
  ads_3A/3 -> [ADS3A_3_ads_3A]  "<ads_3A>"
  [ADS3A_3_ads_3A] -> Self_checking_pair_ADS3A/3(In3)
  ads_3A/2 -> [ADS3A_2_ads_3A]  "<ads_3A>"
  [ADS3A_2_ads_3A] -> Self_checking_pair_ADS3A/2(In2)
  ads_3A/1 -> [ADS3A_1_ads_3A]  "<ads_3A>"
  [ADS3A_1_ads_3A] -> Self_checking_pair_ADS3A/1(In1)
  ads_2B/8 -> [ADS2B_8_ads_2B]  "<ads_2B>"
  [ADS2B_8_ads_2B] -> Self_checking_pair_ADS2B/8(In8)
  ads_2B/7 -> [ADS2B_7_ads_2B]  "<ads_2B>"
  [ADS2B_7_ads_2B] -> Self_checking_pair_ADS2B/7(In7)
  ads_2B/6 -> [ADS2B_6_ads_2B]  "<ads_2B>"
  [ADS2B_6_ads_2B] -> Self_checking_pair_ADS2B/6(In6)
  ads_2B/5 -> [ADS2B_5_ads_2B]  "<ads_2B>"
  [ADS2B_5_ads_2B] -> Self_checking_pair_ADS2B/5(In5)
  ads_2B/4 -> [ADS2B_4_ads_2B]  "<ads_2B>"
  [ADS2B_4_ads_2B] -> Self_checking_pair_ADS2B/4(In4)
  ads_2B/3 -> [ADS2B_3_ads_2B]  "<ads_2B>"
  [ADS2B_3_ads_2B] -> Self_checking_pair_ADS2B/3(In3)
  ads_2B/2 -> [ADS2B_2_ads_2B]  "<ads_2B>"
  [ADS2B_2_ads_2B] -> Self_checking_pair_ADS2B/2(In2)
  ads_2B/1 -> [ADS2B_1_ads_2B]  "<ads_2B>"
  [ADS2B_1_ads_2B] -> Self_checking_pair_ADS2B/1(In1)
  ads_2A/8 -> [ADS2A_8_ads_2A]  "<ads_2A>"
  [ADS2A_8_ads_2A] -> Self_checking_pair_ADS2A/8(In8)
  ads_2A/7 -> [ADS2A_7_ads_2A]  "<ads_2A>"
  [ADS2A_7_ads_2A] -> Self_checking_pair_ADS2A/7(In7)
  ads_2A/6 -> [ADS2A_6_ads_2A]  "<ads_2A>"
  [ADS2A_6_ads_2A] -> Self_checking_pair_ADS2A/6(In6)
  ads_2A/5 -> [ADS2A_5_ads_2A]  "<ads_2A>"
  [ADS2A_5_ads_2A] -> Self_checking_pair_ADS2A/5(In5)
  ads_2A/4 -> [ADS2A_4_ads_2A]  "<ads_2A>"
  [ADS2A_4_ads_2A] -> Self_checking_pair_ADS2A/4(In4)
  ads_2A/3 -> [ADS2A_3_ads_2A]  "<ads_2A>"
  [ADS2A_3_ads_2A] -> Self_checking_pair_ADS2A/3(In3)
  ads_2A/2 -> [ADS2A_2_ads_2A]  "<ads_2A>"
  [ADS2A_2_ads_2A] -> Self_checking_pair_ADS2A/2(In2)
  ads_2A/1 -> [ADS2A_1_ads_2A]  "<ads_2A>"
  [ADS2A_1_ads_2A] -> Self_checking_pair_ADS2A/1(In1)
  ads_1B/8 -> [ADS1B_8_ads_1B]  "<ads_1B>"
  [ADS1B_8_ads_1B] -> Self_checking_pair_ADS1B/8(In8)
  ads_1B/7 -> [ADS1B_7_ads_1B]  "<ads_1B>"
  [ADS1B_7_ads_1B] -> Self_checking_pair_ADS1B/7(In7)
  ads_1B/6 -> [ADS1B_6_ads_1B]  "<ads_1B>"
  [ADS1B_6_ads_1B] -> Self_checking_pair_ADS1B/6(In6)
  ads_1B/5 -> [ADS1B_5_ads_1B]  "<ads_1B>"
  [ADS1B_5_ads_1B] -> Self_checking_pair_ADS1B/5(In5)
  ads_1B/4 -> [ADS1B_4_ads_1B]  "<ads_1B>"
  [ADS1B_4_ads_1B] -> Self_checking_pair_ADS1B/4(In4)
  ads_1B/3 -> [ADS1B_3_ads_1B]  "<ads_1B>"
  [ADS1B_3_ads_1B] -> Self_checking_pair_ADS1B/3(In3)
  ads_1B/2 -> [ADS1B_2_ads_1B]  "<ads_1B>"
  [ADS1B_2_ads_1B] -> Self_checking_pair_ADS1B/2(In2)
  ads_1B/1 -> [ADS1B_1_ads_1B]  "<ads_1B>"
  [ADS1B_1_ads_1B] -> Self_checking_pair_ADS1B/1(In1)
  ads_1A/8 -> [ADS1A_8_ads_1A]  "<ads_1A>"
  [ADS1A_8_ads_1A] -> Self_checking_pair_ADS1A/8(In8)
  ads_1A/7 -> [ADS1A_7_ads_1A]  "<ads_1A>"
  [ADS1A_7_ads_1A] -> Self_checking_pair_ADS1A/7(In7)
  ads_1A/6 -> [ADS1A_6_ads_1A]  "<ads_1A>"
  [ADS1A_6_ads_1A] -> Self_checking_pair_ADS1A/6(In6)
  ads_1A/5 -> [ADS1A_5_ads_1A]  "<ads_1A>"
  [ADS1A_5_ads_1A] -> Self_checking_pair_ADS1A/5(In5)
  ads_1A/4 -> [ADS1A_4_ads_1A]  "<ads_1A>"
  [ADS1A_4_ads_1A] -> Self_checking_pair_ADS1A/4(In4)
  ads_1A/3 -> [ADS1A_3_ads_1A]  "<ads_1A>"
  [ADS1A_3_ads_1A] -> Self_checking_pair_ADS1A/3(In3)
  ads_1A/2 -> [ADS1A_2_ads_1A]  "<ads_1A>"
  [ADS1A_2_ads_1A] -> Self_checking_pair_ADS1A/2(In2)
  ads_1A/1 -> [ADS1A_1_ads_1A]  "<ads_1A>"
  [ADS1A_1_ads_1A] -> Self_checking_pair_ADS1A/1(In1)
  sidestick2_pitch_1/8 -> [sidestick2_pitch_1_8_sidestick2_pitch_1]  "<sidestick2_pitch_1>"
  [sidestick2_pitch_1_8_sidestick2_pitch_1] -> Self_checking_pair_SSC2_pitch1/8(In8)
  sidestick2_pitch_1/7 -> [sidestick2_pitch_1_7_sidestick2_pitch_1]  "<sidestick2_pitch_1>"
  [sidestick2_pitch_1_7_sidestick2_pitch_1] -> Self_checking_pair_SSC2_pitch1/7(In7)
  sidestick2_pitch_1/6 -> [sidestick2_pitch_1_6_sidestick2_pitch_1]  "<sidestick2_pitch_1>"
  [sidestick2_pitch_1_6_sidestick2_pitch_1] -> Self_checking_pair_SSC2_pitch1/6(In6)